simulink model "MCVT_Integration"  (R2020a)
settings: Solver=ode8  StartTime=0.0  StopTime=T_end  FixedStep=dt  RelTol=1e-3  EnableMultiTasking=on
values from neighbouring files (symbols this model uses):
  assumed_power_voltage = 1.25e2    (= 125)    [MCVT_Input_File.m]    (also in MCVT_Run_QuickCheck.m)
  battery_power_toggle = 1    [MCVT_Input_File.m]    (also in MCVT_Run_QuickCheck.m)
  battery_trickle_charge_param = 0.1    [MCVT_Input_File.m]    (also in MCVT_Run_QuickCheck.m)
  battery_trickle_leakage_param = 0.01    [MCVT_Input_File.m]    (also in MCVT_Run_QuickCheck.m)
  damage_case_id = 3    [MCVT_Input_File.m]    (also in MCVT_Run_QuickCheck.m = 1)
  dt = 1/fs    (= 0.001)    [MCVT_Run_QuickCheck.m]    (also in MCVT_Sim_Set.m)
  dt_2 = 1/fs_2    (= 0.001)    [MCVT_Run_QuickCheck.m]    (also in MCVT_Sim_Set.m)
  dt_3 = 1/fs_3    (= 0.001)    [MCVT_Run_QuickCheck.m]    (also in MCVT_Sim_Set.m)
  dt_5 = 1/fs_5    (= 0.005)    [MCVT_Run_QuickCheck.m]    (also in MCVT_Sim_Set.m)
  dt_8 = 1/fs_8    (= 0.05)    [MCVT_Run_QuickCheck.m]    (also in MCVT_Sim_Set.m)
  dt_9 = 1/fs_9    (= 0.001)    [MCVT_Run_QuickCheck.m]    (also in MCVT_Sim_Set.m)
  dust_met_duration = 3    [MCVT_Input_File.m]    (also in MCVT_Run_QuickCheck.m = 2)
  ECLSS_indoor_set_temperature = 298.15    [MCVT_Input_File.m]    (also in MCVT_Run_QuickCheck.m)
  energy_store_max_o = 150    [MCVT_Input_File.m]    (also in MCVT_Run_QuickCheck.m)
  energy_store_o = 120    [MCVT_Input_File.m]    (also in MCVT_Run_QuickCheck.m)
  fs = 1000    [MCVT_Run_QuickCheck.m]    (also in MCVT_Sim_Set.m)
  gain_charge_param = 1    [MCVT_Input_File.m]    (also in MCVT_Run_QuickCheck.m)
  ground_temperature = 373    [MCVT_Habitat_Design_Input_File.m]    (also in MCVT_Run_QuickCheck.m)
  I_max_charge = 800    [MCVT_Input_File.m]    (also in MCVT_Run_QuickCheck.m)
  I_max_discharge = 800    [MCVT_Input_File.m]    (also in MCVT_Run_QuickCheck.m)
  initial_pressure = 1e5    (= 100000)    [MCVT_Input_File.m]    (also in MCVT_Run_QuickCheck.m)
  initial_temperature = 295    [MCVT_Input_File.m]    (also in MCVT_Run_QuickCheck.m)
  interior_leak_rate = 0.00415    [MCVT_Input_File.m]    (also in MCVT_Run_QuickCheck.m)
  launch_dust_accum_start_time = 10    [MCVT_Input_File.m]    (also in MCVT_Run_QuickCheck.m = 2)
  launch_dust_accum_stop_time = 12    [MCVT_Input_File.m]    (also in MCVT_Run_QuickCheck.m = 3)
  meteorite_impact_time = 2    [MCVT_Input_File.m]    (also in MCVT_Run_QuickCheck.m = 1)
  nuclear_power_toggle = 1    [MCVT_Input_File.m]    (also in MCVT_Run_QuickCheck.m)
  print_interval = 30    [MCVT_Input_File.m]
  SOC_lower_thres = 0.5    [MCVT_Input_File.m]    (also in MCVT_Run_QuickCheck.m)
  SOC_upper_thres = 0.9    [MCVT_Input_File.m]    (also in MCVT_Run_QuickCheck.m)
  solar_power_toggle = 1    [MCVT_Input_File.m]    (also in MCVT_Run_QuickCheck.m)
  space_temperature = 4    [MCVT_Habitat_Design_Input_File.m]    (also in MCVT_Run_QuickCheck.m)
  Start_angle = 90    [MCVT_Input_File.m]    (also in MCVT_Run_QuickCheck.m)
undefined symbols (not defined in the file or in its folder): A_flow_restrictor, ACTIVITY_LOOKUP_TABLE, air, Aref, Bmap, Bmap2, Bmap3, comms, DiscIntNeverNeededParam, DmgSPL, dust_parameters, ECLSS_dust_damage_repair_time, ECLSS_heater_capacity, ECLSS_IPS_restrictor, ECLSS_IPS_setpointPressure, ECLSS_paint_damage_repair_time, ECLSS_radiatorLoop_discreteNumber, ECLSS_Vst, emptyActivity, expected_heat_add, FI, habitat, HIEM2SMM, HIEM2STM, HIEMDamageElements, impact_numbering, initial_SPLT_damage_index, input_air, input_Cond, input_CP, input_discretization, input_EV, input_Evap, input_radiatorLoop, K_ECLSS_dust_single, K_ECLSS_paint_single, K_Structure_damage_single, Kref, kW_to_W, meteoroidDustDep, mFRF, ndelays, nominalDustDep, noverlap, nuclear_toggle_gain, output_limits, panel_paint0, Ps0, reference_temperature, RepairTime_ECLSS_factor, Sensor_Location_SMM, SMM_T0, SMM_T1, SMM_T2, SMM_T3, SMM_T4, SMM_T4_0, SMM_T4_1, SMM_T4_2, solar_FDD_K ... (+73)
libraries: neural x350, simulink x39, xpcutilitieslib x7, xpcrtudplib x7, dspstat3 x1
(part 38 of 43)

== matlab function MCVT_Integration/Sys5 - ECLSS/Active Thermal Control System (ATCS)/ATCS ==
  data: X output; Pe output; Pc output; Tfs output; Trs output; Tf_hp_in output size=1; Tf_hp_out output size=1; Vair_out output; Tair_out output size=1; WCP output; Yc output; Xk input; Vop input; input_discretization input; input_Evap input; input_Cond input; input_CP input; input_air input; input_RL input; input_dust input; Fs input; Tair_in input; Vair_in input; nCP input; input_EV input; Ye output
function [X, Pe, Pc, Tfs,Trs, Tf_hp_in, Tf_hp_out, Vair_out, Tair_out, WCP, Yc, Ye] = Active_Thermal_Control_system(Xk, Vop, input_discretization, input_Evap, input_Cond, input_CP,  input_air, input_RL, input_dust, Fs, Tair_in, Vair_in, nCP, input_EV)
%% Documentation
% Purpose: Solve state variables for heat pump cycle
% Date Created: 20 June 2020
% Date Last Modified: 27 July 2021
% Modeler Name: Jaewon Park, CJ Pan (Purdue)
% Funding Acknowledgement: Funded by the NASA RETHi Project (80NSSC19K1076)

% Version Number: MCVT v1.4

% Subsystem Connections: receives input from subsystem 9.

% INPUTS %
%   - Xk: state variables from previous time step, [1x111]
%   - Vop: condensor managing coefficient, type: float
%   - Fs: solar irradiation, unit: W/m^2, type: float
%   - input_discretization: discretization information, [1x4]
%   - input_Evap: evaporator parameters, [1x3]
%   - input_Cond: condensor parameters, [1x3]
%   - input_CP: compressor parameters, [1x2]
%   - input_air: air parameters, [1x2]
%   - input_RL: radiator loop parameters, [1x12]
%   - input_dust: subsection panel parameters, [1x151]
%   - nCP: compressor motor speed, unit: Hz, type: float
%   - Tair_in: air flow temperature through evaporator, unit: C, type: float
%   - Vair_in: air flow volumetric flow rate through evaporator, unit:
%   m^3/s, type: float
%   - input_EV: Expansion valve parameters, [1x2]
 
% OUTPUTS %
%   - X: state variables, [1x111]
%   - Tfs: temperature of HTF, unit: C, type: float
%   - Trs: temperature of radiator, unit: C, type: float
%   - Tf_hp_in: HTF temperature at the condensor inlet, unit: C, type: float
%   - Tf_hp_out: HTF temperature at the condesnor outlet, unit: C, type: float
%   - Pe: evaporator pressure, unit: bar, type: float
%   - Pc: condensor pressure, unit: bar, type: float
%   - WCP: compressor power consumption, unit: kW, type: float
%   - Vair_out: volumetric flow rate of air circulation, unit: m^3/s, type: float
%   - Tair_out: air temperature at the evaporator outlet, unit: C, type: float
%   - Yc: liquid volume fraction in condensor, type: float
%   - Ye: liquid volume fraction in evaporator, type: float

% Function Dependencies: no dependency

% No Data Depenencies
%% Solving process

%%
% X: state variables
% Tf_hp_in:  Inlet temperature of NH3 in the radiator loop to the condenser, unit:C
% Tf_hp_out: Outlet temperature of the NH3 going through the condenser, unit:C
% WCP:   Compressor power consumption, unit:KW
% Vair_out: Indoor air flowrate through the evaporator,unit:m^3/s
% Tair_out: Air temperature after going through the evaporator, unit:m^3
% Ye:       Refrigerant liquid volume fraction in the evaporator,unit:-

%% 
Nhp=input_discretization(3);         % Number of variables in the heat pump loop;
N=input_discretization(4);           % Discrete points of the HTF flow channel of the radiator loop.
Nt=Nhp+2*N-1;

%%
% Maximum number of iterations
Max_iter=10;
X=Xk;

for iter=1:Max_iter
    
    X1=X;
    
    [f, J]=coupled_system_model(X1,Xk,input_discretization, input_Evap, input_Cond, input_CP,  input_EV, input_air,input_RL, input_dust,Fs, nCP,Vair_in,Tair_in,Vop);
   
   %% Newton-Raphson method
   %Stoping creterion for Newton iteration
   err=0;
   for k=1:Nt
       err=err+abs(f(k));
   end

   if err<0.0001
      break
   else  
     
  % Newton-Raphson iteration
    temp=J\f;
    X=X1-temp;
   end
 
% End of Newton iteration loop
end


 %% Outputs
 Tair_out=X(1);                      % Evaporator air temperature
 Pe=X(3);                            % Evaporator pressure
 Ye=X(4);                            % Evaporator liquid fraction
 Pc=X(5);                            % Condensor pressure
 Yc=X(6);                            % Condensor liquid fraction
 Tf_hp_out=X(7);                     % NH3 outlet temperature from the condenser
 mCP=X(10);                          % Compressor mass flow rate
 Tf_hp_in=X(12);                     % NH3 inlet temperature to the condenser
%% Airflow rate through the evaporator
 Vair_out=Vair_in;

 %% Compressor power consumption:
 if Pc<Pe
     alpha=1.05;
 else
     alpha=Pc/Pe;   
 end
 eta_is=SVR_eff_is(alpha); 
 hve=SVR_hv(Pe)*1000;          % Compressor inlet enthalpy, unit: J/kg
 hvc=SVR_hv(Pc)*1000;
 hCP=1/eta_is*abs((hvc-hve))+hve;   % Compressor outlet enthalpy, unit: J/kg
 WCP=mCP*(hCP-hve)/1000;       % Compressor power consumption, unit: kW
 %% Sesnor data
 N_sensors=6;     % Number of sensors;
 
 dNs=floor((N-1)/(N_sensors+1));
 
 
 Tfs=zeros(1,N_sensors);
 Trs=zeros(1,N_sensors);
 
 
 for i=1:N_sensors
     
   Tfs(i)=X(Nhp+2+dNs*(i-1));
   Trs(i)=X(Nhp+N+2+dNs*(i-1));
 
 end
 
 

end




function  [fval, J]=coupled_system_model(X,Xk, input_discretization, input_Evap, input_Cond, input_CP, input_EV,input_air, input_RL,input_dust,Fs,nCP, Vair_in, Tair_in,Vop)

%% State variables
%  X=[Ta, xEV, Pe, Ye, Pc, Yc, Thp_out, me,  mc, mCP, mEV, Thp_in,Tf,Tr]

%% Outputs
% Function values: fval
% Jacobian matrix: J

%% Inputs:


%% computational input parameters
dt=input_discretization(1);          % Discrete time step;
dz=input_discretization(2);          % Length of a finite volume of the radiator loop;
Nhp=input_discretization(3);         % Number of variables in the heat pump loop;
N=input_discretization(4);           % Discrete points of the HTF flow channel of the radiator loop.

% Initilization
J=zeros(Nhp+2*N-1,Nhp+2*N-1);
fval=zeros(Nhp+2*N-1,1);


%% Variables
Ta=X(1);                         % Habitat interior temperature, unit: C
xEV=X(2);                        % Outlet expansion valve vapor mass quality 
Pe=X(3);                         % Evaporation pressure,    unit: bar
Ye=X(4);                         % Liquid phase volume fraction
Pc=X(5);                         % Condensing pressure,     unit: bar
Yc=X(6);                         % Vapor phase volume fraction
Thp_out=X(7);                    % Outlet temperature of the HTF in the radiator loop from the condenser, unit: C
me=X(8);                         % Evaporated refrigerant mass in the evaporator
mc=X(9);                         % Condensed refrigerant mass in the condensor
mCP=X(10);                       % Mass flowrate of refrigerant through the compressor, unit:kg/s
mEV=X(11);                       % Mass flowrate of refrigerant through the Expansion valve, unit:kg/s
Thp_in=X(12);                    % HTF inlet temperature in the radiator loop to the condenser     

Tf=zeros(N);
Tr=zeros(N-1);
Tf(1:N)=X(Nhp+1:Nhp+N);          % HTF  temperature in the radiator loop
Tr(1:N-1)=X(Nhp+N+1:Nhp+2*N-1);  % Radiator fin surface temperature


%% State variables at the previous time step
Pek=Xk(3);
Yek=Xk(4);
Pck=Xk(5);
Yck=Xk(6);
Tfk=Xk(Nhp+1:Nhp+N);
Trk=Xk(Nhp+N+1:Nhp+2*N-1);

%% Parameters for evaporator
Ae=input_Evap(1);
Ue=input_Evap(2);
Ve=input_Evap(3);

%% Parameters for condenser
Ac=input_Cond(1);
Uc=input_Cond(2)*Vop;
Vc=input_Cond(3);

%% Parameters for the compressor

eta_v=input_CP(1);
VD=input_CP(2);


%% Parameters for the expansion valve
cEV=input_EV(1);
aEV=input_EV(2);

%% Parameters of air properties:
rou_air=input_air(1);
cp_air=input_air(2);
% Vair_in=input_air(3);
% Tair_in=input_air(4);

%% Parameters for the radiator loop
Mf=input_RL(1);                       % Total mass flow rate of the HTF in the radiator loop
cpf=input_RL(2);
rouf=input_RL(3);
uf=input_RL(4);
hcf=input_RL(5);
r=input_RL(6);
w=input_RL(7);
l=input_RL(8);
kr=input_RL(9);
rour=input_RL(10);
cpr=input_RL(11);
eta_fin=input_RL(12);

%% Dust and thermal control paint properties
% Dust conamination output
% input_dust=zeros(1,2+2*N);
% input_dust(1)=delta;
% input_dust(2)=kd;
% input_dust(3:N+2)=epsilon;
% input_dust(N+3:2*N+2)=alpha;
kd=input_dust(1);
delta=input_dust(2:N+1);
epsilon=input_dust(N+2:2*N+1);
alpha=input_dust(2*N+2:3*N+1);



%% Solar radiation parameters
Botz=5.67*10^(-8);  

%% Parameter evaulations for the HP system
Ce_min=Vair_in*rou_air*cp_air;
Cc_min=Mf*cpf;
NTUe=Ue*Ae/(Ce_min);
NTUc=Uc*Ac/(Cc_min);
epsilon_e=1-exp(-NTUe);
epsilon_c=1-exp(-NTUc);


%% Functions:
% Enthalpy, unit: kJ/kg
hle=SVR_hl(Pek)*1000;           
hve=SVR_hv(Pek)*1000;                 
         
hvc=SVR_hv(Pck)*1000;   
hlc=SVR_hl(Pck)*1000;

ulc=SVR_ul(Pck)*1000;
uvc=SVR_uv(Pck)*1000;
ule=SVR_ul(Pek)*1000;
uve=SVR_uv(Pek)*1000;

dulc=SVR_dul(Pck)*1000;
duvc=SVR_duv(Pck)*1000;
dule=SVR_dul(Pek)*1000;
duve=SVR_duv(Pek)*1000;

% Densitis and their gradients with respect to pressure
rou_ve=SVR_rou_v(Pek);
rou_vc=SVR_rou_v(Pck);
rou_lc=SVR_rou_l(Pck);
rou_le=SVR_rou_l(Pek);
drou_ve=SVR_drou_v(Pek);
drou_vc=SVR_drou_v(Pck);
drou_le=SVR_drou_l(Pek);
drou_lc=SVR_drou_l(Pck);

% Saturated Temperature, unit: C
Te=SVR_Ts(Pek);        
Tc=SVR_Ts(Pck);
dTe=SVR_dTs(Pek);
dTc=SVR_dTs(Pck);
       
 %% Air temperature coming from the evaporator*****************************
 % X=[Ta, xEV, Pe, Xe, Pc, Xc, Thp_out, me,  mc, mCP, mEV,Thp_in, Tf, Tr]
 
   fval(1,1)=(Tair_in-Ta)-epsilon_e*(Tair_in-Te);
   
   J(1,1)=-1;
   J(1,2)=0;
   J(1,3)=epsilon_e*dTe*(Pe-Pek)/dt;
   J(1,4)=0;
   J(1,5)=0;
   J(1,6)=0;
   J(1,7)=0;
   J(1,8)=0;
   J(1,9)=0;
   J(1,10)=0;
   J(1,11)=0;
         
 %% Vapor mass quality after the expansion vavle***************************
 % X=[Ta, xEV, Pe, Xe, Pc, Xc, Thp_out, me,  mc, mCP, mEV,Thp_in, Tf, Tr]
   fval(2,1)=xEV*(hve-hle)-(hlc-hle);
   
   J(2,1)=0;
   J(2,2)=hve-hle;
   J(2,3)=0;
   J(2,4)=0;
   J(2,5)=0;
   J(2,6)=0;
   J(2,7)=0;
   J(2,8)=0;
   J(2,9)=0;
   J(2,10)=0;
   J(2,11)=0;
 
 %% Evpaorator model*******************************************************
 
    %% Total Energy balance inside the evaporator
    % X=[Ta, xEV, Pe, Xe, Pc, Xc, Thp_out, me,  mc, mCP, mEV,Thp_in, Tf, Tr]
    
    A1=Ve*rou_le*ule*(Ye-Yek)/dt;
    A2=Ve*rou_le*Ye*dule*(Pe-Pek)/dt;
     
    B1=-Ve*rou_ve*uve*(Ye-Yek)/dt;
    B2=Ve*(1-Ye)*rou_ve*duve*(Pe-Pek)/dt;
    B3=Ve*(1-Ye)*uve*drou_ve*(Pe-Pek)/dt;
    %
    fval(3,1)=A1+A2+B1+B2+B3-epsilon_e*Ce_min*(Tair_in-Te)-mEV*hlc+mCP*hve;
    %
    J(3,1)=0;
    J(3,2)=0;
    J(3,3)=Ve*rou_le*Ye*dule/dt+Ve*(1-Ye)*rou_ve*duve/dt+Ve*(1-Ye)*uve*drou_ve/dt+epsilon_e*Ce_min*dTe*(Pe-Pek)/dt;
    J(3,4)=Ve*rou_le*ule/dt+Ve*rou_le*dule*(Pe-Pek)/dt-Ve*rou_ve*uve/dt-Ve*rou_ve*duve*(Pe-Pek)/dt-Ve*uve*drou_ve*(Pe-Pek)/dt;
    J(3,5)=0;
    J(3,6)=0;
    J(3,7)=0;
    J(3,8)=0;
    J(3,9)=0;
    J(3,10)=hve;
    J(3,11)=-hlc;
    
    %% Mass balance of the vapor phase in the evaporator:
    % X=[Ta, xEV, Pe, Xe, Pc, Xc, Thp_out, me,  mc, mCP, mEV,Thp_in, Tf, Tr]
    C1=Ve*(1-Ye)*drou_ve*(Pe-Pek)/dt;
    C2=-Ve*rou_ve*(Ye-Yek)/dt;
    
    fval(4,1)=C1+C2-me+mCP-xEV*mEV;
    
    J(4,1)=0;
    J(4,2)=-mEV;
    J(4,3)=Ve*(1-Ye)*drou_ve/dt;
    J(4,4)=-Ve*drou_ve*(Pe-Pek)/dt-Ve*rou_ve/dt;
    J(4,5)=0;
    J(4,6)=0;
    J(4,7)=0;
    J(4,8)=-1;
    J(4,9)=0;
    J(4,10)=1;
    J(4,11)=-xEV;
  
   %% Mass balance of the liquid phase in the evaporator:
   % X=[Ta, xEV, Pe, Xe, Pc, Xc, Thp_out, me,  mc, mCP, mEV,Thp_in, Tf, Tr]
    fval(5,1)=Ve*rou_le*(Ye-Yek)/dt+Ve*Ye*drou_le*(Pe-Pek)/dt+me-(1-xEV)*mEV;
    
    J(5,1)=0;
    J(5,2)=mEV;
    J(5,3)=Ve*Ye*drou_le/dt;
    J(5,4)=Ve*rou_le/dt+Ve*drou_le*(Pe-Pek)/dt;
    J(5,5)=0;
    J(5,6)=0;
    J(5,7)=0;
    J(5,8)=1;
    J(5,9)=0;
    J(5,10)=0;
    J(5,11)=-(1-xEV);
       
 %% Condenser model********************************************************
 
    %% Mass balance of the vapor phase in the condenser:
    % X=[Ta, xEV, Pe, Xe, Pc, Xc, Thp_out, me,  mc, mCP, mEV,Thp_in, Tf, Tr]
     D1=Vc*(1-Yc)*drou_vc*(Pc-Pck)/dt;
     D2=-Vc*rou_vc*(Yc-Yck)/dt;
     fval(6,1)=D1+D2+mc-mCP;
     
     J(6,1)=0;
     J(6,2)=0;
     J(6,3)=0;
     J(6,4)=0;
     J(6,5)=Vc*(1-Yc)*drou_vc/dt;
     J(6,6)=-Vc*drou_vc*(Pc-Pck)/dt-Vc*rou_vc/dt;
     J(6,7)=0;
     J(6,8)=0;
     J(6,9)=1;
     J(6,10)=-1;
     J(6,11)=0;
      
   %% Mass balance of the liquid phase in the condenser:
   % X=[Ta, xEV, Pe, Xe, Pc, Xc, Thp_out, me,  mc, mCP, mEV,Thp_in, Tf, Tr]
     fval(7,1)=Vc*rou_lc*(Yc-Yck)/dt+Vc*Yc*drou_lc*(Pc-Pck)/dt-mc+mEV;
     
     J(7,1)=0;
     J(7,2)=0;
     J(7,3)=0;
     J(7,4)=0;
     J(7,5)=Vc*Yc*drou_lc/dt;
     J(7,6)=Vc*rou_lc/dt+Vc*drou_lc*(Pc-Pck)/dt;
     J(7,7)=0;
     J(7,8)=0;
     J(7,9)=-1;
     J(7,10)=0;
     J(7,11)=1;
       
   %% Energy balance of the whole condenser:
   % X=[Ta, xEV, Pe, Xe, Pc, Xc, Thp_out, me,  mc, mCP, mEV,Thp_in, Tf, Tr]
     D1=Vc*rou_lc*ulc*(Yc-Yck)/dt;
     D2=Vc*rou_lc*Yc*dulc*(Pc-Pck)/dt;
     
     E1=-Vc*rou_vc*uvc*(Yc-Yck)/dt;
     E2=Vc*(1-Yc)*rou_vc*duvc*(Pc-Pck)/dt;
     E3=Vc*(1-Yc)*uvc*drou_vc*(Pc-Pck)/dt;
     
     
     ap=Pck/Pek;
  %  eta_i0=eff_isentropic_spline(alpha);       % Isentropic efficiency
     eta_is=SVR_eff_is(ap);                  % Isentropic efficiency
%    eta_is=0.6;
     
     hCP=1/eta_is*(hvc-hve)+hve;
      
     fval(8,1)=D1+D2+E1+E2+E3-epsilon_c*Cc_min*(Thp_in-Tc)-mCP*hCP+mEV*hlc;
          
     J(8,1)=0;
     J(8,2)=0;
     J(8,3)=0;
     J(8,4)=0;
     
     J(8,5)=Vc*rou_lc*Yc*dulc/dt+Vc*(1-Yc)*rou_vc*duvc/dt+Vc*(1-Yc)*uvc*drou_vc/dt+epsilon_c*Cc_min*dTc*(Pc-Pck)/dt;
     
     J(8,6)=Vc*rou_lc*ulc/dt+Vc*rou_lc*dulc*(Pc-Pck)/dt-Vc*rou_vc*uvc/dt-Vc*rou_vc*duvc*(Pc-Pck)/dt-Vc*uvc*drou_vc*(Pc-Pck)/dt;
     
     J(8,7)=0;
     J(8,8)=0;
     J(8,9)=0;
     
     J(8,10)=-hCP;
     J(8,11)=hlc;
     J(8,Nhp)=-epsilon_c*Cc_min;
     
   %% Energy balance of the HTF
   % X=[Ta, xEV, Pe, Xe, Pc, Xc, Thp_out, me,  mc, mCP, mEV,Thp_in, Tf, Tr]
     fval(9,1)=Thp_out-Thp_in-epsilon_c*(Tc-Thp_in);
     
     J(9,1)=0;
     J(9,2)=0;
     J(9,3)=0;
     J(9,4)=0;
     J(9,5)=-epsilon_c*(dTc)*(Pc-Pck)/dt;
     J(9,6)=0;
     J(9,7)=1;
     J(9,8)=0;
     J(9,9)=0;
     J(9,10)=0;
     J(9,11)=0;
     J(9,Nhp)=epsilon_c;
 
 %% Compressor model*****************************************************************************************************
 % X=[Ta, xEV, Pe, Ye, Pc, Yc, Thp_out, me,  mc, mCP, mEV,Thp_in, Tf, Tr]

  fval(10)=eta_v*nCP*rou_ve*VD-mCP;
   
  J(10,1)=0;
  J(10,2)=0;      
  J(10,3)=eta_v*nCP*drou_ve*(Pe-Pek)/dt*VD;
  J(10,4)=0;
  J(10,5)=0;
  J(10,6)=0;
  J(10,7)=0;
  J(10,8)=0;
  J(10,9)=0;
  J(10,10)=-1;
  J(10,11)=0;

          
 %% Expansion vavle model***************************************************************
 
   fval(11,1)=mEV-cEV*aEV*(rou_lc*(Pc-Pe)^2)^(0.25);
    
   J(11,1)=0;
   J(11,2)=0;
   J(11,3)=-cEV*aEV*(rou_lc)^(0.5)*0.25*((Pc-Pe)^2)^(-0.75)*(-2)*(Pc-Pe);
   J(11,4)=0;     
   J(11,5)=-cEV*aEV*(rou_lc)^0.5*0.25*((Pc-Pe)^2)^(0.75)*2*(Pc-Pe);
   J(11,6)=0;
   J(11,7)=0;
   J(11,8)=0;
   J(11,9)=0;
   J(11,10)=0;
   J(11,11)=1;

    
 %% Node equation at the radiator loop inlet and the HP system outlet*******************
    fval(Nhp,1)=Tf(1)-Thp_out;
    
    J(Nhp,7)=-1;
    J(Nhp,Nhp+1)=1;
    
 %% HTF equations in the radiator loop*************************************************** 
    H1=zeros(N-1,1);
    H2=zeros(N-1,1);
    a2=zeros(N,1);
    
    a1=rouf*cpf*pi*r^2;
    for i=1:N
      a2(i)=1/(2*pi*r*hcf)+1/(2*l)*(delta(i)/kd+w/kr);
    end
    
    for i=1:N-1
        
        H1(i)=a1*((0.5*(Tf(i+1)+Tf(i))-0.5*(Tfk(i+1)+Tfk(i)))/dt+uf*(Tf(i+1)-Tf(i))/dz);
        H2(i)=(eta_fin*Tr(i)-0.5*eta_fin*(Tf(i+1)+Tf(i)))/a2(i);   
        
        fval(Nhp+i,1)=H1(i)-H2(i);
   
        J(Nhp+i,Nhp+i)=a1*(0.5/dt-uf/dz)+0.5*eta_fin/a2(i);
        J(Nhp+i,Nhp+i+1)=a1*(0.5/dt+uf/dz)+0.5*eta_fin/a2(i);
        J(Nhp+i,Nhp+N+i)=-1/a2(i);   
        
    end
    
    
  %% Node equation at the radiator loop outlet and HP system inlet***********************
    fval(Nhp+N,1)=Tf(N)-Thp_in;
    
    J(Nhp+N,Nhp)=-1;
    J(Nhp+N,Nhp+N)=1;
     
  %% Equations for the radiator surface fin temperature***********************************
    P1=zeros(N-1,1);
    P2=zeros(N-1,1);
    b2=zeros(N,1);
    
    b1=rour*cpr*(4*w*l-pi*r^2)/(2*l);
    for i=1:N
        b2(i)=l/(pi*r)*1/hcf+delta(i)/kd+w/kr;
    end
   
    for i=1:N-1
        
        P1(i)=b1*(Tr(i)-Trk(i))/dt;
        P2(i)=(0.5*eta_fin*(Tf(i+1)+Tf(i))-Tr(i))/b2(i)-epsilon(i)*Botz*(Tr(i)+273)^4+alpha(i)*Fs;
        
        fval(Nhp+N+i,1)=P1(i)-P2(i);
        
        J(Nhp+N+i,Nhp+i)  =-0.5*eta_fin/b2(i);
        J(Nhp+N+i,Nhp+i+1)=-0.5*eta_fin/b2(i);
        J(Nhp+N+i,Nhp+N+i)=b1/dt+1/b2(i)+epsilon(i)*Botz*4*(Tr(i)+273)^3;
              
    end
        
  %%*****************************************************************************************  
     
end





function fval=SVR_hl(x)

     b0=216.9047;
     U=[-147.011537341731,91.2095458143568,100.377528506695,-2.94070733693279,-68.6277243647794,-38.8388153707651,30.4433500569375,56.7488272175872,22.5221002860706,-21.5822292836131,-25.6571946012934,6.58380204955175,33.5378280620784,28.0436179185491,2.76321423232039,-11.6086109396015,-1.64829070742468,18.8165138435085,27.6234884761579,17.2211936938004,-0.0624548045987238,-5.53598624617724,7.47250615630266,26.2610290879764,30.0497524253377,12.0539468970891,-9.38927946635403,-7.34194561515610,21.5572599976047,46.2097526452955,32.7175601179956,-10.8811238732925,-33.3975721468835,2.74982129193261,66.4364881063497,78.2028454331051,1.12984625475030,-91.6666759199630,-56.6473719284788,198.123547154558];
     XX=[0.500000000000000;1;1.50000000000000;2;2.50000000000000;3;3.50000000000000;4;4.50000000000000;5;5.50000000000000;6;6.50000000000000;7;7.50000000000000;8;8.50000000000000;9;9.50000000000000;10;10.5000000000000;11;11.5000000000000;12;12.5000000000000;13;13.5000000000000;14;14.5000000000000;15;15.5000000000000;16;16.5000000000000;17;17.5000000000000;18;18.5000000000000;19;19.5000000000000;20];
     %%
     K=RBF_kernel_hl(x,XX');
     fval=K*U'+b0;

end
function K=RBF_kernel_hl(A,B)
    %--------------------------------------
    delta=1.6;
    r=abs(A'-B);
    K=exp(-r.^2./(2*delta^2));
    
end

%%
function fval=SVR_hv(x)

 b0=370.899734122625;
 U=[-40.9273591680419,-21.5935125659206,-5.59112953749365,6.79356190478178,15.4622339071023,20.5063252929680,22.1971431798735,20.9647615201377,17.3671142070421,5.71067856872314,-7.31594327670373,-16.9184653137379,-16.0924750302601,11.8098290478805,27.4592149631120,14.0706063778731,-2.73690999170973,-0.550225738823883,13.9121626361286,21.6635097986220,13.8236669357833,-0.495238584738499,-1.18605829102167,18.3017732160671,32.6586689089617,13.0431602949852,-21.2521206598578,-9.16852526571096,77.8419671984454];
 XX=[0.200000000000000;0.300000000000000;0.400000000000000;0.500000000000000;0.600000000000000;0.700000000000000;0.800000000000000;0.900000000000000;1;1.20000000000000;1.40000000000000;1.60000000000000;2;2.50000000000000;3;3.50000000000000;4;4.50000000000000;5;5.50000000000000;6;6.50000000000000;7;7.50000000000000;8;8.50000000000000;9;9.50000000000000;10];
%%

 K=RBF_kernel_hv(x,XX');
 fval=K*U'+b0;


end
function K=RBF_kernel_hv(A,B)
    %--------------------------------------
    delta=1.2;
    
    r=abs(A'-B);
    K=exp(-r.^2./(2*delta^2));
    %---------------------------------------

end

%%
function fval=SVR_ul(x)


     b0=193.0724;
     U=[-136.905957841766,62.0638264129271,102.888307349165,37.0622269424511,-84.3105579941704,16.3551562151247,71.6519564688184,-3.65144356934930,-4.94774266977309,37.5560630821606,22.6346080294351,18.9736511565362,24.8310658956324,6.38539956721357,35.2333782802201,54.7590549502640,-16.3829359731759,-0.137029301156915,115.351722725590,21.3905643723024,-133.106640306290,212.214843494837];
     XX=[0.500000000000000;1;1.50000000000000;2;3;4;5;6;7;8;9;10;11;12;13;14;15;16;17;18;19;20];

     K=RBF_kernel_ul(x,XX');
     fval=K*U'+b0;
 

end
function K=RBF_kernel_ul(A,B)

    %--------------------------------------
    delta=2.0;
    r=abs(A'-B);
    K=exp(-r.^2./(2*delta^2));
    
    
end

%%
function fval=SVR_uv(x)

 
 b0=362.9372;
 U=[-64.4627868896218,33.0286954344370,51.7329276097141,18.1354429838109,-40.7398353402888,11.2519158956231,37.5650319263164,-0.961655494967726,-0.627478427265556,20.8559448963694,12.5749799321496,10.5761373367731,13.9283729194019,4.66646922169418,18.4910709780448,28.0932823501881,-6.92355226828981,0.918622920131555,57.5782987588697,11.1508736961507,-64.9651039013168,105.164373443551];
 XX=[0.500000000000000;1;1.50000000000000;2;3;4;5;6;7;8;9;10;11;12;13;14;15;16;17;18;19;20];

 %%
 K=RBF_kernel_uv(x,XX');
 fval=K*U'+b0;


end
function K=RBF_kernel_uv(A,B)

    %--------------------------------------
    delta=2.0;
    r=abs(A'-B);
    K=exp(-r.^2./(2*delta^2));
    
    %---------------------------------------
end

%%
function fval=SVR_dul(x)

 b0=63.5613;
 U=[30.7321787225233,-34.7682041680216,-39.6648233329658,-8.89286573261098,29.9536471059340,-18.4780949260904,-35.6304835858768,0.117229650043993,1.91059211680961,-22.3170874970404,-20.3230210095034,-2.30761768776214,-3.19389037122530,-23.8817186111946,-21.6766380364430,8.74142295020969,-4.63876784760920,-47.8474312350974,-6.82921455135653,50.4055246710633,-84.3549192459257];
 XX=[1;1.50000000000000;2;2.50000000000000;3.50000000000000;4.50000000000000;5.50000000000000;6.50000000000000;7.50000000000000;8.50000000000000;9.50000000000000;10.5000000000000;11.5000000000000;12.5000000000000;13.5000000000000;14.5000000000000;15.5000000000000;16.5000000000000;17.5000000000000;18.5000000000000;19.5000000000000];

%%
K=RBF_kernel_dul(x,XX');
fval=K*U'+b0;

%%

end
function K=RBF_kernel_dul(A,B)

    %--------------------------------------
    delta=2.0;
    r=abs(A'-B);
    K=exp(-r.^2./(2*delta^2));
    
  %---------------------------------------
end

%%
function fval=SVR_duv(x)

 b0=34.2339;
 U=[17.6210438164122,-19.0890554962105,-22.1689938233803,-5.22237621117989,16.8135810713597,-9.86151540620288,-19.8870302423362,-0.0337521269943475,1.37141348692438,-12.2422720777981,-11.4320724566521,-1.22508584890361,-1.52773808600230,-13.2306167975409,-12.1210031967530,4.95789435242796,-2.45312090131685,-26.5782731409338,-3.77050901930001,28.0211121469759,-46.7655530945912];
 XX=[1;1.50000000000000;2;2.50000000000000;3.50000000000000;4.50000000000000;5.50000000000000;6.50000000000000;7.50000000000000;8.50000000000000;9.50000000000000;10.5000000000000;11.5000000000000;12.5000000000000;13.5000000000000;14.5000000000000;15.5000000000000;16.5000000000000;17.5000000000000;18.5000000000000;19.5000000000000];

%%
K=RBF_kernel_duv(x,XX');
fval=K*U'+b0;

%%

end
function K=RBF_kernel_duv(A,B)
    %--------------------------------------
    delta=2.0;
    r=abs(A'-B);
    K=exp(-r.^2./(2*delta^2));

end

%%
function fval=SVR_rou_v(x)

%  
 b0=61.0019;
 U=[-110.010405464022,-60.0176416768082,-19.1832735051432,11.8107665788528,32.8589180260900,44.4181700524238,47.4557695927075,43.3628006137010,33.8406248120022,20.7691748472074,6.06728782801554,-8.44441016549827,-21.1680879484563,-30.8320650845715,-36.5661221193007,-37.9442260006044,-34.9926043328899,-28.1635764959692,-18.2778614843816,-6.44010491596116,6.06598728539356,17.8953087541584,27.7634578231486,34.5646708654101,37.4771850454806,36.0483378885541,30.2528961883220,20.5197442646310,7.72409753278001,-6.85524855284618,-21.6116035127525,-34.7144442780600,-44.2349683859507,-48.2843299256177,-45.1544390053976,-33.4506676732067,-12.2061410299929,19.0314800943630,60.1477726661629,110.448228174211];
 XX=[0.500000000000000;1;1.50000000000000;2;2.50000000000000;3;3.50000000000000;4;4.50000000000000;5;5.50000000000000;6;6.50000000000000;7;7.50000000000000;8;8.50000000000000;9;9.50000000000000;10;10.5000000000000;11;11.5000000000000;12;12.5000000000000;13;13.5000000000000;14;14.5000000000000;15;15.5000000000000;16;16.5000000000000;17;17.5000000000000;18;18.5000000000000;19;19.5000000000000;20];
 
%%
K=RBF_kernel_rou_v(x,XX');
fval=K*U'+b0;

%%

end
function K=RBF_kernel_rou_v(A,B)

    %--------------------------------------
    delta=5.5;
    
    r=abs(A'-B);
    K=exp(-r.^2./(2*delta^2));
       
    %---------------------------------------

end
%%
function fval=SVR_drou_v(x)


 b0=6.2878;
 U=[-0.645600496319800,0.00280710647285763,-0.128523185090695,-0.371059825771556,-0.249040449419433,-0.145262740346941,-0.261534711708063,-0.289635585013218,-0.195018362225185,-0.204350062153497,-0.263316472355448,-0.227838361490364,-0.186776216609973,-0.214968869112473,-0.223969501844191,-0.190903486464387,-0.181288905398566,-0.188169292713347,-0.179916472711878,-0.168670002679263,-0.155691337424898,-0.146438740826721,-0.150134832861951,-0.130428151321952,-0.105255024562754,-0.128958495695105,-0.115344816703605,-0.0439425332626478,-0.0821154090684979,-0.136893733995876,0.000722395644727099,0.0434138383351717,-0.160065506329906,-0.0694570093147260,0.253212212485578,-0.0220300872366157,-0.411097205312408,0.560731521840678];
 XX=[1;1.50000000000000;2;2.50000000000000;3;3.50000000000000;4;4.50000000000000;5;5.50000000000000;6;6.50000000000000;7;7.50000000000000;8;8.50000000000000;9;9.50000000000000;10;10.5000000000000;11;11.5000000000000;12;12.5000000000000;13;13.5000000000000;14;14.5000000000000;15;15.5000000000000;16;16.5000000000000;17;17.5000000000000;18;18.5000000000000;19;19.5000000000000];

%%
K=RBF_kernel_drou_v(x,XX');

fval=K*U'+b0;


end
function K=RBF_kernel_drou_v(A,B)
    %--------------------------------------
    delta=1.0;
    r=abs(A'-B);
    K=exp(-r.^2./(2*delta^2));
    
end


%%
function fval=SVR_rou_l(x)


 b0=1444.89843435874;
 U=[428.723962033338,-274.041822934414,-257.453398878137,62.9224459610305,203.469797618099,56.1962705412696,-128.259528430837,-123.969491347213,14.1934056798791,84.5547403857168,16.8822021979032,-73.2549870779955,-71.0514036996334,-4.99590070906891,23.5840208177016,-12.7323304329617,-49.9742265089649,-39.6607471146850,-11.5336425356760,-14.3542725622210,-39.1310420343584,-40.3354900862689,-14.4566141248448,-9.24092008746084,-45.2182714712866,-71.6581830360650,-37.5011959870718,18.5931760308582,6.21266095373688,-80.3376058997672,-125.074148167455,-41.4893387540001,77.7882260275590,51.5900887410329,-131.549787657516,-237.664381077510,-62.7293338209629,236.887289945919,190.892210656241,-546.582911491639];
 XX=[0.500000000000000;1;1.50000000000000;2;2.50000000000000;3;3.50000000000000;4;4.50000000000000;5;5.50000000000000;6;6.50000000000000;7;7.50000000000000;8;8.50000000000000;9;9.50000000000000;10;10.5000000000000;11;11.5000000000000;12;12.5000000000000;13;13.5000000000000;14;14.5000000000000;15;15.5000000000000;16;16.5000000000000;17;17.5000000000000;18;18.5000000000000;19;19.5000000000000;20];
 
%%
K=RBF_kernel_rou_l(x,XX');
fval=K*U'+b0;

%%


end
function K=RBF_kernel_rou_l(A,B)

    %--------------------------------------
    delta=1.5;
    
    r=abs(A'-B);
    K=exp(-r.^2./(2*delta^2));
    
end

function fval=SVR_drou_l(x)

 b0=-166.9984;
 U=[-102.428586709673,119.818921994468,129.472532123064,23.2567948972211,-71.4512378186433,-75.6874139289568,0.352543492937891,81.5589380930246,96.2063413985549,34.8553782804110,-44.9839839225240,-69.7973237270008,-13.9442880641230,76.0206359122559,117.370779780088,60.7080949198072,-60.2017110361847,-136.910003724967,-49.0739253874437,260.382411006638];
 XX=[1;1.50000000000000;2;2.50000000000000;3;3.50000000000000;4;4.50000000000000;5;5.50000000000000;6;6.50000000000000;7;7.50000000000000;8;8.50000000000000;9;9.50000000000000;10;10.5000000000000];

%%
K=RBF_kernel_drou_l(x,XX');

fval=K*U'+b0;


end

function K=RBF_kernel_drou_l(A,B)

    %--------------------------------------
    delta=2.0;
    r=abs(A'-B);
    K=exp(-r.^2./(2*delta^2));
    
end



%%
function fval=SVR_Ts(x)

 b0=-17.3377160329879;
 U=[-83.5588090591053,-39.5349163520128,-4.42322132162465,20.8906905874919,36.2508282107436,42.2274996033668,40.0493785005812,31.4767348933012,18.6279276615444,-10.8618137275603,-32.0863399797709,-35.1275504499911,8.17487216632950,54.7910052220241,18.6122759267098,-12.1113853250976,18.4708737161559,40.3331418121612,15.1464147112551,0.184594095092861,27.8909348607504,42.6467518540924,9.35285437588132,-3.97396383716234,45.5910118193790,60.6056251010551,-16.8612070725385,-35.1411517759882,140.725541833844];
 XX=[0.200000000000000;0.300000000000000;0.400000000000000;0.500000000000000;0.600000000000000;0.700000000000000;0.800000000000000;0.900000000000000;1;1.20000000000000;1.40000000000000;1.60000000000000;2;2.50000000000000;3;3.50000000000000;4;4.50000000000000;5;5.50000000000000;6;6.50000000000000;7;7.50000000000000;8;8.50000000000000;9;9.50000000000000;10];

%%
K=RBF_kernel_Ts(x,XX');

fval=K*U'+b0;

end


function K=RBF_kernel_Ts(A,B)

    %--------------------------------------
    delta=1.0;
    %---------------------------------------
    r=abs(A'-B);
    K=exp(-r.^2./(2*delta^2));
     
end

%%
function fval=SVR_dTs(x)

 b0=71.8358;
 U=[43.2253753951252,-44.4866977517640,-53.0760393445871,-16.8027177449529,19.5201314572328,27.3344815165684,7.23047558600656,-19.2209358159734,-30.2593498870455,-20.5611233891047,-2.09239580218645,8.73257643152745,5.06650931522573,-6.73067266050005,-15.0653277180566,-14.0063763503380,-7.22839827037227,-2.65315614511877,-4.09588779412739,-8.18878285672250,-8.98507277301715,-4.97061533813828,-1.04317708379614,-3.24457839107947,-11.5196542426476,-18.2282329390293,-14.9037215930196,-1.47626104546741,11.2550296966832,9.83962920716502,-8.87375414008906,-31.6770650839250,-37.1150020568324,-13.5206879980983,26.5238310632875,47.6672940143698,10.3938264996776,-104.668814630376];
 XX=[1;1.50000000000000;2;2.50000000000000;3;3.50000000000000;4;4.50000000000000;5;5.50000000000000;6;6.50000000000000;7;7.50000000000000;8;8.50000000000000;9;9.50000000000000;10;10.5000000000000;11;11.5000000000000;12;12.5000000000000;13;13.5000000000000;14;14.5000000000000;15;15.5000000000000;16;16.5000000000000;17;17.5000000000000;18;18.5000000000000;19;19.5000000000000];

%%
K=RBF_kernel_dTs(x,XX');
fval=K*U'+b0;

%%

end
function K=RBF_kernel_dTs(A,B)
    delta=2.;
    
    r=abs(A'-B);
    K=exp(-r.^2./(2*delta^2));
    
end




function fval=SVR_eff_is(x)

 
 b0=-1.33572545906389;
 U=[-1.67955547822396,-0.773410048042453,-0.0325841794240679,0.548998818228278,1.26996169388123,1.46920813159106,1.36933703816769,0.967354204714020,-0.227331765130889,-1.20230600988890,-1.41111143546160,-0.272131703827964,1.42707438697242,1.51930462604316,-0.866540756238048,0.563145943675950,1.22249692077683,-0.453339709460247,0.971418096181273,0.486234308904801,0.101363112978364,0.955676831170538,-0.0353513940812759,0.716038338168739,0.698980490045480,-0.483171050083914,1.13004808834272,0.883576535593288,-1.20857226125922,0.863193742599757,2.30142851309483,-1.59660821531808,-2.34233008416595,3.94851732445928];
 XX=[1.13966480446927;1.19180633147114;1.24394785847300;1.29608938547486;1.40037243947859;1.47858472998138;1.58286778398510;1.68715083798883;1.89571694599628;2.10428305400372;2.31284916201117;2.62569832402235;2.96461824953445;3.38175046554935;3.98137802607076;4.65921787709497;5.41527001862197;6.11918063314711;6.79702048417132;7.55307262569832;8.30912476722533;8.96089385474860;9.71694599627560;10.4208566108007;11.0465549348231;11.6983240223464;12.3500931098696;12.9497206703911;13.5232774674115;14.1229050279330;14.6443202979516;15.1657355679702;15.6610800744879;15.9739292364991];

%%
K=RBF_kernel_eff_is(x,XX');

fval=K*U'+b0;

%%

end


function K=RBF_kernel_eff_is(A,B)
   
    delta=1.2;
    r=abs(A'-B);
    K=exp(-r.^2./(2*delta^2));
    
end

== matlab function MCVT_Integration/Sys 6 - Health Management and Agents/Decision Maker/Simulink Communication Modules/Structure_Dmg//MCVT API to HMS/UDP_Send/MATLAB Function1 ==
  data: SizeInfo output; signal input
function SizeInfo=size_Info(signal)

info=size(signal);

SizeInfo=[info(1), info(2)];

== matlab function MCVT_Integration/Sys 6 - Health Management and Agents/Decision Maker/Simulink Communication Modules/Agent//MCVT API to HMS/UDP_Send_C2/MATLAB Function ==
  data: hi0 output; len input; hdr_Info input; rowcol input; len0 output
function [hi0, len0]=headerInfo(len, hdr_Info, rowcol)
src=hdr_Info(1);
dest=hdr_Info(2);
type=hdr_Info(3);
prio=hdr_Info(4);
row=rowcol(1); 
col=rowcol(2);
% len=0; 
hi0=[src, dest, type, prio, row, col];
len0=len;
% hi=uint8(hi);

== matlab function MCVT_Integration/Sys 6 - Health Management and Agents/Decision Maker/Simulink Communication Modules/Agent//MCVT API to HMS/UDP_Send_C2/MATLAB Function1 ==
  data: SizeInfo output; signal input
function SizeInfo=size_Info(signal)

info=size(signal);

SizeInfo=[info(1), info(2)];

== matlab function MCVT_Integration/Sys 6 - Health Management and Agents/Decision Maker/Simulink Communication Modules/Agent//MCVT API from HMS/MATLAB Function ==
  data: u input; y output; Receive_length input
function y = fcn(u, Receive_length)
    if Receive_length ~=0
        y = u;
    else
        y = -1;
    end
end

== matlab function MCVT_Integration/Timer/MATLAB Function ==
  data: clock input; print_interval input
function time_display(clock, print_interval)
    if mod(clock, print_interval) == 0 && clock >= print_interval 
        fprintf('Simulation Time: %0.2f sec\n', clock);
    end
end

== matlab function MCVT_Integration/Sys8 - Interior Environment/Habitat Interior Environment Model/C2K ==
  data: C input; K output
function K = TempConversion(C)

K = C + 273.15;

end

== matlab function MCVT_Integration/Sys2 - Structural System/Sys2_Structural_System/Fault Detection and Diagnosis (FDD) 2.3/Accelerometer/Accelerometer/Accelerometer/Maximum//minimum Output Voltage ==
  data: u input; y output
function y = fcn(u)

y = u(1:9);

if u(1:9) > 17
    y = 17*ones(9,1);
else
    if u(1:9) < 7
        y = 7*ones(9,1);
    end
end

== matlab function MCVT_Integration/Sys9 - Exterior Environment/Exterior Environment/Mapping function from Solar Irradiation to SPLT and STM ==
  data: pos_out input; xyz_SR input; SR_STM output; Sb_tot input; SR_STM_ei_th output
function [SR_STM,SR_STM_ei_th] = Mapping(pos_out,xyz_SR,Sb_tot)
% %% Mapping of Solar Radiation provided by SR model to other models

%XYZ coordinates provided by the original SR model%
x_o=xyz_SR(:,1); %Original x-coordinate, m%
y_o=xyz_SR(:,2); %Original y-coordinate, m%
z_o=xyz_SR(:,3); %Original z-coordinate, m%

%XYZ coordinates provided by the user to supply to STM model%
x_f=pos_out(:,1); %Final x-coordinate, m%
y_f=pos_out(:,2); %Final y-coordinate, m%
z_f=pos_out(:,3); %Final z-coordinate, m%

%Mapping using pdist() function%
SR2OM = zeros(size(x_f,1),1); %Initialization%

for i=1:size(x_f,1)
    dxyz = nan(size(x_o,1),1);
    for j=1:size(x_o,1)
        dxyz_SR = [pos_out(i,:) ; xyz_SR(j,:)];
        dxyz(j,1) = pdist(dxyz_SR,'euclidean');
    end
    [a,b] = min(dxyz); %'a' gives the distance value and the 'b' provides the index%
    SR2OM(i) = Sb_tot(b);
%     Ind(i,1)=b;
end

SR_STM = SR2OM; %SR_STM =Solar Radiation to the STM model%
SR_STM_mat=reshape(SR_STM,10,6);
SR_STM_ei_th=0;%Initialization%
SR_STM_ei_th=SR_STM_mat(8,3);


end

== matlab function MCVT_Integration/Sys2 - Structural System/Sys2_Structural_System/Fault Detection and Diagnosis (FDD) with Binary Classifer/FRF from Accelerometer 3/FRF Calculation ==
  data: exc input; frf output; acc input; m input; noverlap input; w input
function frf = xfunc(exc,acc,m,noverlap,w)

%  [XFER21,FREQV,P] = XFUNC(X,Y,FS,M,NOVERLAP) will estimate the
%	complex transfer function given time histories of the
%	input, X, and the output, Y. Given the complex response,
%	the magnitude and phase can be determined by using the
%	ABS and ANGLE functions. FS is the sampling frequency (Hz)
%	at which the data was taken. The X and Y sequences of
%	N points are divided into NS sections of M points each
%	(M must be a power of two). Using an M-point FFT,
%	successive sections are Hanning windowed, FFT'd and
%	averaged.	** SOME PARTS FROM SPECTRUM FUNCTION
%
%	XFUNC(X,Y,FS) 	--> NO AVERAGING
%	XFUNC(X,Y,FS,M)	--> AVERAGING BUT NO OVERLAP
%	NO OUTPUT ARGUMENTS WILL CREATE A BODE PLOT
%
% 	The output P is the matrix of power spectrums in the order
%	P = [Pxx,Pyy,Pxy].
%
%	NOTES: 	Xfer data may be written to a specified file.
%		Variables were chosen to immitate certain IP names.
%		Depending on the anti-aliasing filter used a certain
%			percentage of the data will include aliased
%			data (IP is 78% correct).

% COMMENTS
% nargin = 3 --> no overlap, no averaging
% nargin = 4 --> no overlap, averaging data
% nargin = 5 --> some overlap, and averaging data
% set color variable to easily change plot color
% may also write data to a file

frf = zeros(1,256*3);
x = exc;

for k = 1:1:3
    t = k+6;
    y = acc(:,t);
    
%     color	= 'b';
%     
%     if (nargin == 3), m = length(y); noverlap = 0; fmax = fs/2; end
%     if (nargin == 4)
%         if (length(y) == 1)
%             noverlap = m;
%             m = length(y);
%         else
%             noverlap = 0;
%         end
%         fmax = fs/2;
%     end
    %keyboard
    x 	= x(:);		% Make sure x and y are column vectors
    y 	= y(:);
    
    % DEFINE CONSTANTS
     n 	= max(size(x));	% Number of data points in entire time history
     ns 	= fix((n-noverlap)/(m-noverlap));	% Number of samples (frames)
    % (k = fix(n/m) for noverlap=0)
%     w 	= hann(m);	% hanning is replaced by hann
    % Type of windowing: change this for application
    % (Try HAMMING, BLACKMAN, BARTLETT, or your own)
    % HANNING is best for narrow-band random !!
     KMU 	= ns*norm(w)^2;	% Norm. scale factor (AVERAGING & WINDOWING)
     index 	= 1:m;
    % fprintf(1,['\n%5i',' Samples Averaged\n'],ns)
    
    
    % FIND POWER SPECTRUMS OF EACH SAMPLE AND SUM
    Pxx = zeros(m,1)+0i; 
%     Pyy = Pxx; 
    Pxy = Pxx; % Plus 0i
    for i = 1:ns
        xw 	= w.*x(index);		% detrend? no difference
        yw 	= w.*y(index);
        index 	= index + (m - noverlap);
        Xx 	= fft(xw);
        Yy 	= fft(yw);
        Pxx 	= Pxx + Xx.*conj(Xx);
%         Pyy 	= Pyy + Yy.*conj(Yy);
        Pxy 	= Pxy + Yy.*conj(Xx);
    end
    
    % SELECT
    %select = [1:m/2];
    select = [1:m/2+1];   % include DC AND Nyquist
    %FreqV	= [fs/2/(m/2):fs/2/(m/2):fs/2]';		% (Nyquist)
%     FreqV	= (select - 1)'*fs/m;
%     mcorrect	= round(.78*max(select));		% first 78%
%     fcorrect	= FreqV(mcorrect);
    
    % AVERAGED & SCALED DATA
    Pxx = Pxx(select)./KMU;			% Double sided
%     Pyy = Pyy(select)./KMU;
    Pxy = Pxy(select)./KMU;
%     P   = [Pxx,Pyy,Pxy];
    
    Xfer21 	= Pxy./Pxx;
    frf(1,256*(k-1)+1:256*k) = abs(Xfer21(1:256,1));
end

% %keyboard
% % NO OUTPUT ARGUMENTS, PLOT BODE
% if (nargout == 0),
%     
%     fig1	= gcf;
%     subplot(211)
%     plot(FreqV,db(Xfer21),color);grid
%     xlabel('Frequency (Hz)')
%     ylabel('Gain (dB)')
%     title('Transfer Function')
%     limits1	= axis;hold on
%     plot([fcorrect,fcorrect],[limits1(3),limits1(4)],'r');
%     xlim([0,fmax]);
%     
%     hold off
%     
%     subplot(212)
%     plot(FreqV,angle(Xfer21)*180/pi,color);grid
%     xlabel('Frequency (Hz)')
%     ylabel('Phase (deg)')
%     limits2	= axis;hold on
%     plot([fcorrect,fcorrect],[limits2(3),limits2(4)],'r');
%     hold off
%     axis([0 fmax -200 50]);
%     %	orient tall;print
%     return % Suppress output
% end

== matlab function MCVT_Integration/Sys2 - Structural System/Sys2_Structural_System/Fault Detection and Diagnosis (FDD) with Binary Classifer/Subsystem/Enabled Subsystem3/MATLAB Function ==
  data: u input; y output; thres input
function y = fcn(u, thres)

n=size(u,2);
toa=zeros(1,n);maxvalue=toa;maxtime=toa;maxEvalue=toa;maxEtime=toa;

for k=1:n
    ind=find(abs(u(:,k))>thres);
    if isempty(ind)
        toa(k)=5000;
    else
        toa(k)=min(ind);
    end
end
toanorm=(toa-min(toa));
idx=find(toanorm>1500);
toanorm(idx)=10;

for k=1:n
    [a,b]=max(abs(u(:,k)));
    maxvalue(k)=a;
    maxtime(k)=b;
    [c,d]=max(abs(hilbert(u(:,k))));
    maxEvalue(k)=c;
    maxEtime(k)=d;
end

y=[toanorm,maxvalue./max(maxvalue),maxtime,maxEvalue./max(maxEvalue),maxtime]';

== matlab function MCVT_Integration/Sys2 - Structural System/Sys2_Structural_System/Fault Detection and Diagnosis (FDD) with Binary Classifer/Subsystem/Information Fusion1 ==
  data: u input; y output; T input; dt input
function y = fcn(u, T, dt)

persistent K k1 reSet prob

if isempty(reSet) || isempty(K)
    reSet=0; K=0;
end

if isempty(prob) || isempty(k1) || reSet==1
    prob=zeros(size(u));
    k1=0;
    reSet=0;
end

if rem(T, dt)==0&&T~=0
    K=K+1;
end

if sum(u)~=0 && (k1==0 || (k1~=0 && (K-k1)>3000))
    prob=double(u);
    k1=K;
end

%{
if K<2000 || (K-k1)<50
    y=zeros(size(u)); reSet=1;
else
    y=prob([5,2,3,4,1]);
end
%}

y=prob;

if K<2000
   y=zeros(size(u)); reSet=1;
end

if (K-k1)>50 && (K-k1)<3000
    y=zeros(size(u));
end

if (K-k1)>=3000
    y=zeros(size(u)); reSet=1;
end


end

== matlab function MCVT_Integration/Sys2 - Structural System/Sys2_Structural_System/Fault Detection and Diagnosis (FDD) with Binary Classifer/Subsystem/Trigger Condition3 ==
  data: thres input; u input; repair input; y output
function y = fcn(thres, u, repair)

y=0;
n=size(u,2);
for i=1:n
    if abs(u(end,i))>thres && max(abs(u(1:end-1,i)))<=thres && repair(1)==0
        y=1; break;
    end
end

end
